simulink library "Renault_Library"  (Simulink 8.2)
settings: Solver=ode3  StartTime=0.0  StopTime=10.0  FixedStep=0.020  RelTol=1e-3
undefined symbols (not defined in the file or in its folder): ABC, AssystemProposal, b, chkval, CoeffA_C, CoeffB_C, CoeffMean_C, ConfTime_C, DiagDelay_C, DividePercent, FailureConfActive_C, FallingEdgeDuration_C, HighLevelThresholdVoltage_C, HighLevelVoltage_C, HighLevelVoltageTunable_C, InitValue_C, InputVoltRangeMax_C, InputVoltRangeMin_C, LowActiveContact_C, LowLevelThresholdVoltage_C, LowLevelVoltage_C, MaxOperatingVoltage_C, MinOperatingVoltage_C, mV2V_conversion, OUT1, OutOfRangeHigh_C, OutOfRangeLow_C, PinSupplySaturationMax, PinSupplySaturationMin, Res1Value_C, Res2Value_C, Res3Value_C, RisingEdgeDuration_C, RSA_CANSignalFailMonitoring, SampleTime_C, service14Table, service19Table, service22Table, service24Table, service28Table, service2ETable, service2FTable, service31Table, service87Table, SizeXStateDID2FTable, SizeXTable14, SizeXTable19, SizeXTable22, SizeXTable24, SizeXTable28, SizeXTable2E, SizeXTable2F, SizeXTable31, SizeXTable87, SizeYStateDID2FTable, SizeYTable14, SizeYTable19, SizeYTable22, SizeYTable24, SizeYTable28 ... (+22)
undefined symbols (not defined in the file): BatteryVoltage_ActivationThreshold_C, FrameStatus_AbsentValue_C, FrameStatus_ConfAbsentValue_C, MemThreshold_C, NAMEOFTHEMODEL_SAMPLE_TIME_CST, NbAbs1_C, NbConfAbs1_C, NbConfPres1_C, NbPres1_C, NOMDUSIGNAL_FaultConfTime_C, NOMDUSIGNAL_FaultRehabTime_C, NOMDUSIGNAL_switchvalue_C, NOMDUSIGNAL_unavailablevalue_C, PeriodFrame1_C, ResetTime_C, WakeUpTime_C
libraries: Renault_Library x112, simulink x75
(part 24 of 32)

== system Renault_Library/Services ECU/Diag/RSA_DiagnosticManagement_v02.00/DIAGNOSTIC_SANCTION_TOP/Service3E/SubFunction_In_Active_Session_Service3E ==
  SESSION : Inport
  DIAG_BUFFER_REQUEST : Inport  Port=2
  BitwiseOperator1 : simulink/Logic and Bit Operations/Bitwise Operator  logicop=AND  UseBitMask=on  BitMask=bin2dec('01111111')  BitMaskRealWorld=Stored Integer
  Constant{,4..6} (4 alike) : Constant  Value=true  OutDataTypeStr=boolean  SampleTime=-1
  Constant1 : Constant  Value=TYP_SESSION_ACTIVE.PROGRAMMING_SESSION  OutDataTypeStr=Inherit: Inherit via back propagation  SampleTime=-1
  Constant12 : Constant  Value=ZeroSubFunctionSession1  OutDataTypeStr=Inherit: Inherit via back propagation  SampleTime=-1
  Constant2 : Constant  Value=TYP_SESSION_ACTIVE.EXTENDED_SESSION  OutDataTypeStr=Inherit: Inherit via back propagation  SampleTime=-1
  Constant21 : Constant  Value=ZeroSubFunctionSession2  OutDataTypeStr=Inherit: Inherit via back propagation  SampleTime=-1
  Constant3 : Constant  Value=TYP_SESSION_ACTIVE.SAFETY_SYSTEM_SESSION  OutDataTypeStr=Inherit: Inherit via back propagation  SampleTime=-1
  Constant{7,33,48,62} (4 alike) : Constant  Value=0  OutDataTypeStr=Inherit: Inherit via back propagation  SampleTime=-1
  Constant36 : Constant  Value=ZeroSubFunctionSession3  OutDataTypeStr=Inherit: Inherit via back propagation  SampleTime=-1
  Constant50 : Constant  Value=ZeroSubFunctionSession4  OutDataTypeStr=Inherit: Inherit via back propagation  SampleTime=-1
  Constant8 : Constant  Value=TYP_SESSION_ACTIVE.DEFAULT_SESSION  OutDataTypeStr=Inherit: Inherit via back propagation  SampleTime=-1
  Logical Operator{1,6,12,17} (4 alike) : Logic  OutDataTypeStr=boolean
  LogicalOperator{,5,12,18} (4 alike) : Logic  OutDataTypeStr=boolean
  LogicalOperator24 : Logic  Operator=OR  Inputs=4  OutDataTypeStr=boolean
  RelationalOperator{1..3,7..10,23..24,33,42..43} (12 alike) : RelationalOperator  Operator===  OutDataTypeStr=boolean  InputSameDT=on
  Selector : Selector  InputPortWidth=4095  IndexOptions=Index vector (dialog)  Indices=[2]  OutputSizes=1
  SUBFUNCTION_SUPPORTED_IN_ACTIVE_SESSION : Outport
  wires:
  Constant8/1 -> RelationalOperator9/2
  SESSION/1 -> RelationalOperator9/1, RelationalOperator1/1, RelationalOperator2/1, RelationalOperator3/1
  Constant1/1 -> RelationalOperator1/2
  Constant2/1 -> RelationalOperator2/2
  Constant3/1 -> RelationalOperator3/2
  Selector/1 -> BitwiseOperator1/1
  DIAG_BUFFER_REQUEST/1 -> Selector/1
  Constant7/1 -> RelationalOperator7/2
  Constant12/1 -> RelationalOperator10/1
  Constant6/1 -> RelationalOperator10/2
  RelationalOperator10/1 -> LogicalOperator/1
  Logical Operator1/1 -> LogicalOperator/2
  RelationalOperator9/1 -> Logical Operator1/2
  RelationalOperator7/1 -> Logical Operator1/1
  Constant48/1 -> RelationalOperator33/2
  Constant36/1 -> RelationalOperator8/1
  Constant4/1 -> RelationalOperator8/2
  RelationalOperator8/1 -> LogicalOperator12/1
  Logical Operator6/1 -> LogicalOperator12/2
  RelationalOperator2/1 -> Logical Operator6/2
  RelationalOperator33/1 -> Logical Operator6/1
  Constant62/1 -> RelationalOperator42/2
  Constant50/1 -> RelationalOperator43/1
  Constant/1 -> RelationalOperator43/2
  RelationalOperator43/1 -> LogicalOperator18/1
  Logical Operator12/1 -> LogicalOperator18/2
  RelationalOperator3/1 -> Logical Operator12/2
  RelationalOperator42/1 -> Logical Operator12/1
  Constant33/1 -> RelationalOperator23/2
  Constant21/1 -> RelationalOperator24/1
  Constant5/1 -> RelationalOperator24/2
  RelationalOperator24/1 -> LogicalOperator5/1
  Logical Operator17/1 -> LogicalOperator5/2
  RelationalOperator1/1 -> Logical Operator17/2
  RelationalOperator23/1 -> Logical Operator17/1
  LogicalOperator24/1 -> SUBFUNCTION_SUPPORTED_IN_ACTIVE_SESSION/1
  BitwiseOperator1/1 -> [Number_Subfunction]
  [Number_Subfunction] -> RelationalOperator7/1
  [Number_Subfunction] -> RelationalOperator23/1
  [Number_Subfunction] -> RelationalOperator33/1
  [Number_Subfunction] -> RelationalOperator42/1
  LogicalOperator5/1 -> LogicalOperator24/2
  LogicalOperator12/1 -> LogicalOperator24/3
  LogicalOperator18/1 -> LogicalOperator24/4
  LogicalOperator/1 -> LogicalOperator24/1

== system Renault_Library/Services ECU/Diag/RSA_DiagnosticManagement_v02.00/DIAGNOSTIC_SANCTION_TOP/Service3E/SubFunction_Supported_Service3E ==
  DIAG_BUFFER_REQUEST : Inport
  BitwiseOperator1 : simulink/Logic and Bit Operations/Bitwise Operator  logicop=AND  UseBitMask=on  BitMask=bin2dec('01111111')  BitMaskRealWorld=Stored Integer
  BitwiseOperator4 : simulink/Logic and Bit Operations/Bitwise Operator  logicop=AND  UseBitMask=on  BitMask=bin2dec('10000000')  BitMaskRealWorld=Stored Integer
  Constant : Constant  Value=0  OutDataTypeStr=Inherit: Inherit via back propagation  SampleTime=-1
  Constant16 : Constant  OutDataTypeStr=Inherit: Inherit via back propagation  SampleTime=-1
  Constant3 : Constant  Value=ZeroSubFunction  OutDataTypeStr=Inherit: Inherit via back propagation  SampleTime=-1
  Constant5 : Constant  Value=true  OutDataTypeStr=boolean  SampleTime=-1
  LogicalOperator : Logic  OutDataTypeStr=boolean
  RelationalOperator : RelationalOperator  Operator===  OutDataTypeStr=boolean  InputSameDT=on
  RelationalOperator16 : RelationalOperator  Operator===  OutDataTypeStr=boolean  InputSameDT=on
  RelationalOperator4 : RelationalOperator  Operator===  OutDataTypeStr=boolean  InputSameDT=on
  Selector : Selector  InputPortWidth=4095  IndexOptions=Index vector (dialog)  Indices=[2]  OutputSizes=1
  ShiftArithmetic3 : ArithShift  BitShiftNumber=7
  SUBFUNCTION_SUPPORTED : Outport
  SUPPRESS_POS_RES : Outport  Port=2
  wires:
  DIAG_BUFFER_REQUEST/1 -> Selector/1
  Constant/1 -> RelationalOperator/2
  Constant3/1 -> RelationalOperator4/1
  Constant5/1 -> RelationalOperator4/2
  RelationalOperator/1 -> LogicalOperator/2
  RelationalOperator4/1 -> LogicalOperator/1
  RelationalOperator16/1 -> SUPPRESS_POS_RES/1
  BitwiseOperator4/1 -> ShiftArithmetic3/1
  ShiftArithmetic3/1 -> RelationalOperator16/1
  Selector/1 -> BitwiseOperator4/1, BitwiseOperator1/1
  Constant16/1 -> RelationalOperator16/2
  BitwiseOperator1/1 -> RelationalOperator/1
  LogicalOperator/1 -> SUBFUNCTION_SUPPORTED/1

== system Renault_Library/Services ECU/Diag/RSA_DiagnosticManagement_v02.00/DIAGNOSTIC_SANCTION_TOP/Service87/Check_Lenght_Message_Service87 ==
  REQ_DATA_NUMBER : Inport
  Constant1 : Constant  Value=2  OutDataTypeStr=Inherit: Inherit via back propagation  SampleTime=-1
  Constant8 : Constant  Value=6  OutDataTypeStr=Inherit: Inherit via back propagation  SampleTime=-1
  LogicalOperator : Logic  OutDataTypeStr=boolean
  RelationalOperator1 : RelationalOperator  OutDataTypeStr=boolean  InputSameDT=on
  RelationalOperator9 : RelationalOperator  Operator=<  OutDataTypeStr=boolean  InputSameDT=on
  LENGTH_MESSAGE_CORRECT : Outport
  wires:
  LogicalOperator/1 -> LENGTH_MESSAGE_CORRECT/1
  RelationalOperator1/1 -> LogicalOperator/2
  RelationalOperator9/1 -> LogicalOperator/1
  Constant1/1 -> RelationalOperator1/2
  Constant8/1 -> RelationalOperator9/2
  REQ_DATA_NUMBER/1 -> RelationalOperator1/1, RelationalOperator9/1

== system Renault_Library/Services ECU/Diag/RSA_DiagnosticManagement_v02.00/DIAGNOSTIC_SANCTION_TOP/Service87/Parameter_Valid_Service87 ==
  DIAG_BUFFER_REQUEST : Inport
  Parameter_service87 : Stateflow chart  TreatAsAtomicUnit=on
  PARAMETER_VALID : Outport
  wires:
  Parameter_service87/1(PARAMETER_VALID) -> PARAMETER_VALID/1
  DIAG_BUFFER_REQUEST/1 -> Parameter_service87/1(DIAG_BUFFER_REQUEST)

== system Renault_Library/Services ECU/Diag/RSA_DiagnosticManagement_v02.00/DIAGNOSTIC_SANCTION_TOP/Service87/SubFunction_In_Active_Session_Service87 ==
  SESSION : Inport
  DIAG_BUFFER_REQUEST : Inport  Port=2
  BitwiseOperator1 : simulink/Logic and Bit Operations/Bitwise Operator  logicop=AND  UseBitMask=on  BitMask=bin2dec('01111111')  BitMaskRealWorld=Stored Integer
  Constant1 : Constant  Value=TYP_SESSION_ACTIVE.PROGRAMMING_SESSION  OutDataTypeStr=Inherit: Inherit via back propagation  SampleTime=-1
  Constant{4..5,9..11,13,15,17..20,22} (12 alike) : Constant  Value=true  OutDataTypeStr=boolean  SampleTime=-1
  Constant12 : Constant  Value=VerifyModeTransitionWithFixedParamaterSession1  OutDataTypeStr=Inherit: Inherit via back propagation  SampleTime=-1
  Constant14 : Constant  Value=VerifyModeTransitionWithSpecificParamaterSession1  OutDataTypeStr=Inherit: Inherit via back propagation  SampleTime=-1
  Constant16 : Constant  Value=TransitionModeSession1  OutDataTypeStr=Inherit: Inherit via back propagation  SampleTime=-1
  Constant2 : Constant  Value=TYP_SESSION_ACTIVE.EXTENDED_SESSION  OutDataTypeStr=Inherit: Inherit via back propagation  SampleTime=-1
  Constant21 : Constant  Value=VerifyModeTransitionWithFixedParamaterSession2  OutDataTypeStr=Inherit: Inherit via back propagation  SampleTime=-1
  Constant23 : Constant  Value=VerifyModeTransitionWithSpecificParamaterSession2  OutDataTypeStr=Inherit: Inherit via back propagation  SampleTime=-1
  Constant25 : Constant  Value=TransitionModeSession2  OutDataTypeStr=Inherit: Inherit via back propagation  SampleTime=-1
  Constant3 : Constant  Value=TYP_SESSION_ACTIVE.SAFETY_SYSTEM_SESSION  OutDataTypeStr=Inherit: Inherit via back propagation  SampleTime=-1
  Constant{6,31,46,60} (4 alike) : Constant  Value=3  OutDataTypeStr=Inherit: Inherit via back propagation  SampleTime=-1
  Constant{32,47,61,65} (4 alike) : Constant  Value=2  OutDataTypeStr=Inherit: Inherit via back propagation  SampleTime=-1
  Constant{7,33,48,62} (4 alike) : Constant  OutDataTypeStr=Inherit: Inherit via back propagation  SampleTime=-1
  Constant36 : Constant  Value=VerifyModeTransitionWithFixedParamaterSession3  OutDataTypeStr=Inherit: Inherit via back propagation  SampleTime=-1
  Constant38 : Constant  Value=VerifyModeTransitionWithSpecificParamaterSession3  OutDataTypeStr=Inherit: Inherit via back propagation  SampleTime=-1
  Constant40 : Constant  Value=TransitionModeSession3  OutDataTypeStr=Inherit: Inherit via back propagation  SampleTime=-1
  Constant50 : Constant  Value=VerifyModeTransitionWithFixedParamaterSession4  OutDataTypeStr=Inherit: Inherit via back propagation  SampleTime=-1
  Constant52 : Constant  Value=VerifyModeTransitionWithSpecificParamaterSession4  OutDataTypeStr=Inherit: Inherit via back propagation  SampleTime=-1
  Constant54 : Constant  Value=TransitionModeSession4  OutDataTypeStr=Inherit: Inherit via back propagation  SampleTime=-1
  Constant8 : Constant  Value=TYP_SESSION_ACTIVE.DEFAULT_SESSION  OutDataTypeStr=Inherit: Inherit via back propagation  SampleTime=-1
  Logical Operator{1..2,5..7,10..13,16..18} (12 alike) : Logic  OutDataTypeStr=boolean
  LogicalOperator{,1..2,5,7..8,12..14,18..20} (12 alike) : Logic  OutDataTypeStr=boolean
  LogicalOperator{4,10,16,22} (4 alike) : Logic  Operator=OR  Inputs=3  OutDataTypeStr=boolean
  LogicalOperator24 : Logic  Operator=OR  Inputs=4  OutDataTypeStr=boolean
  RelationalOperator{1..3,6..12,15..16,20,22..26,30,32..35,39,41..43,45} (28 alike) : RelationalOperator  Operator===  OutDataTypeStr=boolean  InputSameDT=on
  Selector : Selector  InputPortWidth=4095  IndexOptions=Index vector (dialog)  Indices=[2]  OutputSizes=1
  SUBFUNCTION_SUPPORTED_IN_ACTIVE_SESSION : Outport
  wires:
  [Number_Subfunction] -> RelationalOperator41/1
  [Number_Subfunction] -> RelationalOperator39/1
  [Number_Subfunction] -> RelationalOperator42/1
  [Number_Subfunction] -> RelationalOperator32/1
  [Number_Subfunction] -> RelationalOperator30/1
  [Number_Subfunction] -> RelationalOperator33/1
  [Number_Subfunction] -> RelationalOperator22/1
  [Number_Subfunction] -> RelationalOperator20/1
  [Number_Subfunction] -> RelationalOperator23/1
  [Number_Subfunction] -> RelationalOperator6/1
  [Number_Subfunction] -> RelationalOperator45/1
  [Number_Subfunction] -> RelationalOperator7/1
  BitwiseOperator1/1 -> [Number_Subfunction]
  LogicalOperator24/1 -> SUBFUNCTION_SUPPORTED_IN_ACTIVE_SESSION/1
  LogicalOperator4/1 -> LogicalOperator24/1
  LogicalOperator22/1 -> LogicalOperator24/4
  LogicalOperator16/1 -> LogicalOperator24/3
  LogicalOperator10/1 -> LogicalOperator24/2
  RelationalOperator22/1 -> Logical Operator16/1
  RelationalOperator23/1 -> Logical Operator17/1
  Logical Operator16/1 -> LogicalOperator8/2
  Constant31/1 -> RelationalOperator22/2
  RelationalOperator20/1 -> Logical Operator18/1
  Constant32/1 -> RelationalOperator20/2
  RelationalOperator1/1 -> Logical Operator16/2, Logical Operator18/2, Logical Operator17/2
  Logical Operator17/1 -> LogicalOperator5/2
  Logical Operator18/1 -> LogicalOperator7/2
  LogicalOperator5/1 -> LogicalOperator10/1
  LogicalOperator8/1 -> LogicalOperator10/3
  LogicalOperator7/1 -> LogicalOperator10/2
  RelationalOperator16/1 -> LogicalOperator8/1
  Constant25/1 -> RelationalOperator16/1
  Constant13/1 -> RelationalOperator16/2
  RelationalOperator15/1 -> LogicalOperator7/1
  Constant23/1 -> RelationalOperator15/1
  Constant11/1 -> RelationalOperator15/2
  RelationalOperator24/1 -> LogicalOperator5/1
  Constant10/1 -> RelationalOperator24/2
  Constant21/1 -> RelationalOperator24/1
  Constant33/1 -> RelationalOperator23/2
  RelationalOperator41/1 -> Logical Operator11/1
  RelationalOperator42/1 -> Logical Operator12/1
  Logical Operator11/1 -> LogicalOperator20/2
  Constant60/1 -> RelationalOperator41/2
  RelationalOperator39/1 -> Logical Operator13/1
  Constant61/1 -> RelationalOperator39/2
  RelationalOperator3/1 -> Logical Operator11/2, Logical Operator13/2, Logical Operator12/2
  Logical Operator12/1 -> LogicalOperator18/2
  Logical Operator13/1 -> LogicalOperator19/2
  LogicalOperator18/1 -> LogicalOperator22/1
  LogicalOperator20/1 -> LogicalOperator22/3
  LogicalOperator19/1 -> LogicalOperator22/2
  RelationalOperator35/1 -> LogicalOperator20/1
  Constant54/1 -> RelationalOperator35/1
  Constant22/1 -> RelationalOperator35/2
  RelationalOperator34/1 -> LogicalOperator19/1
  Constant52/1 -> RelationalOperator34/1
  Constant20/1 -> RelationalOperator34/2
  RelationalOperator43/1 -> LogicalOperator18/1
  Constant19/1 -> RelationalOperator43/2
  Constant50/1 -> RelationalOperator43/1
  Constant62/1 -> RelationalOperator42/2
  RelationalOperator32/1 -> Logical Operator10/1
  RelationalOperator33/1 -> Logical Operator6/1
  Logical Operator10/1 -> LogicalOperator14/2
  Constant46/1 -> RelationalOperator32/2
  RelationalOperator30/1 -> Logical Operator7/1
  Constant47/1 -> RelationalOperator30/2
  RelationalOperator2/1 -> Logical Operator10/2, Logical Operator7/2, Logical Operator6/2
  Logical Operator6/1 -> LogicalOperator12/2
  Logical Operator7/1 -> LogicalOperator13/2
  LogicalOperator12/1 -> LogicalOperator16/1
  LogicalOperator14/1 -> LogicalOperator16/3
  LogicalOperator13/1 -> LogicalOperator16/2
  RelationalOperator26/1 -> LogicalOperator14/1
  Constant40/1 -> RelationalOperator26/1
  Constant18/1 -> RelationalOperator26/2
  RelationalOperator25/1 -> LogicalOperator13/1
  Constant38/1 -> RelationalOperator25/1
  Constant17/1 -> RelationalOperator25/2
  RelationalOperator8/1 -> LogicalOperator12/1
  Constant15/1 -> RelationalOperator8/2
  Constant36/1 -> RelationalOperator8/1
  Constant48/1 -> RelationalOperator33/2
  RelationalOperator6/1 -> Logical Operator5/1
  RelationalOperator7/1 -> Logical Operator1/1
  Logical Operator5/1 -> LogicalOperator2/2
  Constant6/1 -> RelationalOperator6/2
  RelationalOperator45/1 -> Logical Operator2/1
  Constant65/1 -> RelationalOperator45/2
  RelationalOperator9/1 -> Logical Operator5/2, Logical Operator2/2, Logical Operator1/2
  Logical Operator1/1 -> LogicalOperator/2
  Logical Operator2/1 -> LogicalOperator1/2
  LogicalOperator/1 -> LogicalOperator4/1
  LogicalOperator2/1 -> LogicalOperator4/3
  LogicalOperator1/1 -> LogicalOperator4/2
  RelationalOperator12/1 -> LogicalOperator2/1
  Constant16/1 -> RelationalOperator12/1
  Constant9/1 -> RelationalOperator12/2
  RelationalOperator11/1 -> LogicalOperator1/1
  Constant14/1 -> RelationalOperator11/1
  Constant5/1 -> RelationalOperator11/2
  RelationalOperator10/1 -> LogicalOperator/1
  Constant4/1 -> RelationalOperator10/2
  Constant12/1 -> RelationalOperator10/1
  Constant7/1 -> RelationalOperator7/2
  DIAG_BUFFER_REQUEST/1 -> Selector/1
  Selector/1 -> BitwiseOperator1/1
  Constant3/1 -> RelationalOperator3/2
  Constant2/1 -> RelationalOperator2/2
  Constant1/1 -> RelationalOperator1/2
  SESSION/1 -> RelationalOperator3/1, RelationalOperator2/1, RelationalOperator1/1, RelationalOperator9/1
  Constant8/1 -> RelationalOperator9/2

== system Renault_Library/Services ECU/Diag/RSA_DiagnosticManagement_v02.00/DIAGNOSTIC_SANCTION_TOP/Service87/SubFunction_Supported_Service87 ==
  DIAG_BUFFER_REQUEST : Inport
  BitwiseOperator1 : simulink/Logic and Bit Operations/Bitwise Operator  logicop=AND  UseBitMask=on  BitMask=bin2dec('01111111')  BitMaskRealWorld=Stored Integer
  BitwiseOperator4 : simulink/Logic and Bit Operations/Bitwise Operator  logicop=AND  UseBitMask=on  BitMask=bin2dec('10000000')  BitMaskRealWorld=Stored Integer
  Constant1 : Constant  OutDataTypeStr=Inherit: Inherit via back propagation  SampleTime=-1
  Constant10 : Constant  Value=TransitionMode  OutDataTypeStr=Inherit: Inherit via back propagation  SampleTime=-1
  Constant16 : Constant  OutDataTypeStr=Inherit: Inherit via back propagation  SampleTime=-1
  Constant2 : Constant  Value=2  OutDataTypeStr=Inherit: Inherit via back propagation  SampleTime=-1
  Constant3 : Constant  Value=true  OutDataTypeStr=boolean  SampleTime=-1
  Constant4 : Constant  Value=3  OutDataTypeStr=Inherit: Inherit via back propagation  SampleTime=-1
  Constant5 : Constant  Value=true  OutDataTypeStr=boolean  SampleTime=-1
  Constant6 : Constant  Value=VerifyModeTransitionWithFixedParamater  OutDataTypeStr=Inherit: Inherit via back propagation  SampleTime=-1
  Constant7 : Constant  Value=true  OutDataTypeStr=boolean  SampleTime=-1
  Constant8 : Constant  Value=VerifyModeTransitionWithSpecificParamater  OutDataTypeStr=Inherit: Inherit via back propagation  SampleTime=-1
  LogicalOperator1 : Logic  OutDataTypeStr=boolean
  LogicalOperator2 : Logic  OutDataTypeStr=boolean
  LogicalOperator3 : Logic  OutDataTypeStr=boolean
  LogicalOperator4 : Logic  Operator=OR  Inputs=3  OutDataTypeStr=boolean
  RelationalOperator{1..3,5..7,16} (7 alike) : RelationalOperator  Operator===  OutDataTypeStr=boolean  InputSameDT=on
  Selector : Selector  InputPortWidth=4095  IndexOptions=Index vector (dialog)  Indices=[2]  OutputSizes=1
  ShiftArithmetic3 : ArithShift  BitShiftNumber=7
  SUBFUNCTION_SUPPORTED : Outport
  SUPPRESS_POS_RES : Outport  Port=2
  wires:
  LogicalOperator3/1 -> LogicalOperator4/3
  LogicalOperator2/1 -> LogicalOperator4/2
  LogicalOperator1/1 -> LogicalOperator4/1
  BitwiseOperator1/1 -> RelationalOperator3/1, RelationalOperator2/1, RelationalOperator1/1
  Constant16/1 -> RelationalOperator16/2
  Selector/1 -> BitwiseOperator1/1, BitwiseOperator4/1
  ShiftArithmetic3/1 -> RelationalOperator16/1
  BitwiseOperator4/1 -> ShiftArithmetic3/1
  RelationalOperator16/1 -> SUPPRESS_POS_RES/1
  RelationalOperator3/1 -> LogicalOperator3/2
  LogicalOperator4/1 -> SUBFUNCTION_SUPPORTED/1
  RelationalOperator7/1 -> LogicalOperator3/1
  Constant7/1 -> RelationalOperator7/2
  Constant10/1 -> RelationalOperator7/1
  RelationalOperator6/1 -> LogicalOperator2/1
  RelationalOperator2/1 -> LogicalOperator2/2
  Constant8/1 -> RelationalOperator6/1
  Constant5/1 -> RelationalOperator6/2
  RelationalOperator5/1 -> LogicalOperator1/1
  RelationalOperator1/1 -> LogicalOperator1/2
  Constant6/1 -> RelationalOperator5/1
  Constant3/1 -> RelationalOperator5/2
  Constant4/1 -> RelationalOperator3/2
  Constant2/1 -> RelationalOperator2/2
  Constant1/1 -> RelationalOperator1/2
  DIAG_BUFFER_REQUEST/1 -> Selector/1

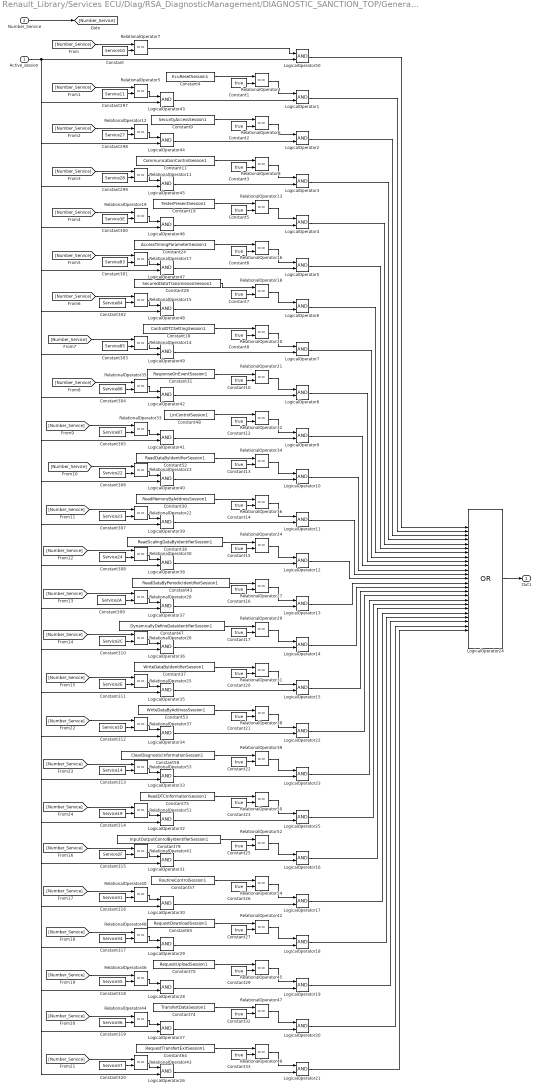
== system Renault_Library/Services ECU/Diag/RSA_DiagnosticManagement/DIAGNOSTIC_SANCTION_TOP/GeneralService/Service_Supported_In_Active_Session/SubsystemDefaultSession ==
  Active_session : Inport
  Number_Service : Inport  Port=2  OutDataTypeStr=uint8
  Constant : Constant  Value=Service10  OutDataTypeStr=Inherit: Inherit via back propagation  SampleTime=-1
  Constant{1..3,5..8,10,12..17,20..23,25..27,29,32..33} (24 alike) : Constant  Value=true  OutDataTypeStr=boolean  SampleTime=-1
  Constant11 : Constant  Value=CommunicationControlSession1  OutDataTypeStr=Inherit: Inherit via back propagation  SampleTime=-1
  Constant18 : Constant  Value=ControlDTCSettingSession1  OutDataTypeStr=Inherit: Inherit via back propagation  SampleTime=-1
  Constant19 : Constant  Value=TesterPresentSession1  OutDataTypeStr=Inherit: Inherit via back propagation  SampleTime=-1
  Constant24 : Constant  Value=AccessTimingParameterSession1  OutDataTypeStr=Inherit: Inherit via back propagation  SampleTime=-1
  Constant28 : Constant  Value=SecuredDataTransmissionSession1  OutDataTypeStr=Inherit: Inherit via back propagation  SampleTime=-1
  Constant297 : Constant  Value=Service11  OutDataTypeStr=Inherit: Inherit via back propagation  SampleTime=-1
  Constant298 : Constant  Value=Service27  OutDataTypeStr=Inherit: Inherit via back propagation  SampleTime=-1
  Constant299 : Constant  Value=Service28  OutDataTypeStr=Inherit: Inherit via back propagation  SampleTime=-1
  Constant30 : Constant  Value=ReadMemoryByAddressSession1  OutDataTypeStr=Inherit: Inherit via back propagation  SampleTime=-1
  Constant300 : Constant  Value=Service3E  OutDataTypeStr=Inherit: Inherit via back propagation  SampleTime=-1
  Constant301 : Constant  Value=Service83  OutDataTypeStr=Inherit: Inherit via back propagation  SampleTime=-1
  Constant302 : Constant  Value=Service84  OutDataTypeStr=Inherit: Inherit via back propagation  SampleTime=-1
  Constant303 : Constant  Value=Service85  OutDataTypeStr=Inherit: Inherit via back propagation  SampleTime=-1
  Constant304 : Constant  Value=Service86  OutDataTypeStr=Inherit: Inherit via back propagation  SampleTime=-1
  Constant305 : Constant  Value=Service87  OutDataTypeStr=Inherit: Inherit via back propagation  SampleTime=-1
  Constant306 : Constant  Value=Service22  OutDataTypeStr=Inherit: Inherit via back propagation  SampleTime=-1
  Constant307 : Constant  Value=Service23  OutDataTypeStr=Inherit: Inherit via back propagation  SampleTime=-1
  Constant308 : Constant  Value=Service24  OutDataTypeStr=Inherit: Inherit via back propagation  SampleTime=-1
  Constant309 : Constant  Value=Service2A  OutDataTypeStr=Inherit: Inherit via back propagation  SampleTime=-1
  Constant31 : Constant  Value=ResponseOnEventSession1  OutDataTypeStr=Inherit: Inherit via back propagation  SampleTime=-1
  Constant310 : Constant  Value=Service2C  OutDataTypeStr=Inherit: Inherit via back propagation  SampleTime=-1
  Constant311 : Constant  Value=Service2E  OutDataTypeStr=Inherit: Inherit via back propagation  SampleTime=-1
  Constant312 : Constant  Value=Service3D  OutDataTypeStr=Inherit: Inherit via back propagation  SampleTime=-1
  Constant313 : Constant  Value=Service14  OutDataTypeStr=Inherit: Inherit via back propagation  SampleTime=-1
  Constant314 : Constant  Value=Service19  OutDataTypeStr=Inherit: Inherit via back propagation  SampleTime=-1
  Constant315 : Constant  Value=Service2F  OutDataTypeStr=Inherit: Inherit via back propagation  SampleTime=-1
  Constant316 : Constant  Value=Service31  OutDataTypeStr=Inherit: Inherit via back propagation  SampleTime=-1
  Constant317 : Constant  Value=Service34  OutDataTypeStr=Inherit: Inherit via back propagation  SampleTime=-1
  Constant318 : Constant  Value=Service35  OutDataTypeStr=Inherit: Inherit via back propagation  SampleTime=-1
  Constant319 : Constant  Value=Service36  OutDataTypeStr=Inherit: Inherit via back propagation  SampleTime=-1
  Constant320 : Constant  Value=Service37  OutDataTypeStr=Inherit: Inherit via back propagation  SampleTime=-1
  Constant37 : Constant  Value=WriteDataByIdentifierSession1  OutDataTypeStr=Inherit: Inherit via back propagation  SampleTime=-1
  Constant38 : Constant  Value=ReadScalingDataByIdentifierSession1  OutDataTypeStr=Inherit: Inherit via back propagation  SampleTime=-1
  Constant4 : Constant  Value=EcuResetSession1  OutDataTypeStr=Inherit: Inherit via back propagation  SampleTime=-1
  Constant43 : Constant  Value=ReadDataByPeriodicIdentifierSession1  OutDataTypeStr=Inherit: Inherit via back propagation  SampleTime=-1
  Constant47 : Constant  Value=DynamicallyDefineDataIdentifierSession1  OutDataTypeStr=Inherit: Inherit via back propagation  SampleTime=-1
  Constant48 : Constant  Value=LinControlSession1  OutDataTypeStr=Inherit: Inherit via back propagation  SampleTime=-1
  Constant52 : Constant  Value=ReadDataByIdentifierSession1  OutDataTypeStr=Inherit: Inherit via back propagation  SampleTime=-1
  Constant53 : Constant  Value=WriteDataByAddressSession1  OutDataTypeStr=Inherit: Inherit via back propagation  SampleTime=-1
  Constant57 : Constant  Value=RoutineControlSession1  OutDataTypeStr=Inherit: Inherit via back propagation  SampleTime=-1
  Constant58 : Constant  Value=ClearDiagnosticInformationSession1  OutDataTypeStr=Inherit: Inherit via back propagation  SampleTime=-1
  Constant64 : Constant  Value=RequestTransfertExitSession1  OutDataTypeStr=Inherit: Inherit via back propagation  SampleTime=-1
  Constant65 : Constant  Value=RequestDownloadSession1  OutDataTypeStr=Inherit: Inherit via back propagation  SampleTime=-1
  Constant70 : Constant  Value=RequestUploadSession1  OutDataTypeStr=Inherit: Inherit via back propagation  SampleTime=-1
  Constant74 : Constant  Value=TransfertDataSession1  OutDataTypeStr=Inherit: Inherit via back propagation  SampleTime=-1
  Constant75 : Constant  Value=ReadDTCInformationSession1  OutDataTypeStr=Inherit: Inherit via back propagation  SampleTime=-1
  Constant79 : Constant  Value=InputOutputConrolByIdentifierSession1  OutDataTypeStr=Inherit: Inherit via back propagation  SampleTime=-1
  Constant9 : Constant  Value=SecurityAccessSession1  OutDataTypeStr=Inherit: Inherit via back propagation  SampleTime=-1
  LogicalOperator{1..23,25..50} (49 alike) : Logic  OutDataTypeStr=boolean
  LogicalOperator24 : Logic  Operator=OR  Inputs=25  OutDataTypeStr=boolean
  RelationalOperator{4..8,11..54} (49 alike) : RelationalOperator  Operator===  OutDataTypeStr=boolean  InputSameDT=on
  Out1 : Outport
  wires:
  Constant/1 -> RelationalOperator7/2
  Active_session/1 -> LogicalOperator33/2, LogicalOperator32/2, LogicalOperator31/2, LogicalOperator30/2, LogicalOperator29/2, LogicalOperator28/2, LogicalOperator27/2, LogicalOperator26/2, LogicalOperator34/2, LogicalOperator40/2, LogicalOperator39/2, LogicalOperator38/2, LogicalOperator37/2, LogicalOperator36/2, LogicalOperator35/2, LogicalOperator41/2, LogicalOperator42/2, LogicalOperator49/2, LogicalOperator48/2, LogicalOperator47/2, LogicalOperator46/2, LogicalOperator45/2, LogicalOperator44/2, LogicalOperator43/2, LogicalOperator50/2
  RelationalOperator7/1 -> LogicalOperator50/1
  [Number_Service] -> RelationalOperator7/1
  Constant297/1 -> RelationalOperator5/2
  Constant4/1 -> RelationalOperator4/1
  Constant1/1 -> RelationalOperator4/2
  RelationalOperator4/1 -> LogicalOperator1/1
  LogicalOperator43/1 -> LogicalOperator1/2
  RelationalOperator5/1 -> LogicalOperator43/1
  [Number_Service] -> RelationalOperator5/1
  Constant298/1 -> RelationalOperator12/2
  Constant9/1 -> RelationalOperator6/1
  Constant2/1 -> RelationalOperator6/2
  RelationalOperator6/1 -> LogicalOperator2/1
  LogicalOperator44/1 -> LogicalOperator2/2
  RelationalOperator12/1 -> LogicalOperator44/1
  [Number_Service] -> RelationalOperator12/1
  Constant299/1 -> RelationalOperator11/2
  Constant11/1 -> RelationalOperator8/1
  Constant3/1 -> RelationalOperator8/2
  RelationalOperator8/1 -> LogicalOperator3/1
  LogicalOperator45/1 -> LogicalOperator3/2
  RelationalOperator11/1 -> LogicalOperator45/1
  [Number_Service] -> RelationalOperator11/1
  Constant300/1 -> RelationalOperator19/2
  Constant19/1 -> RelationalOperator13/1
  Constant5/1 -> RelationalOperator13/2
  RelationalOperator13/1 -> LogicalOperator4/1
  LogicalOperator46/1 -> LogicalOperator4/2
  RelationalOperator19/1 -> LogicalOperator46/1
  [Number_Service] -> RelationalOperator19/1
  Constant301/1 -> RelationalOperator17/2
  Constant24/1 -> RelationalOperator16/1
  Constant6/1 -> RelationalOperator16/2
  RelationalOperator16/1 -> LogicalOperator5/1
  LogicalOperator47/1 -> LogicalOperator5/2
  RelationalOperator17/1 -> LogicalOperator47/1
  [Number_Service] -> RelationalOperator17/1
  Constant302/1 -> RelationalOperator15/2
  Constant28/1 -> RelationalOperator18/1
  Constant7/1 -> RelationalOperator18/2
  RelationalOperator18/1 -> LogicalOperator6/1
  LogicalOperator48/1 -> LogicalOperator6/2
  RelationalOperator15/1 -> LogicalOperator48/1
  [Number_Service] -> RelationalOperator15/1
  Constant303/1 -> RelationalOperator14/2
  Constant18/1 -> RelationalOperator20/1
  Constant8/1 -> RelationalOperator20/2
  RelationalOperator20/1 -> LogicalOperator7/1
  LogicalOperator49/1 -> LogicalOperator7/2
  RelationalOperator14/1 -> LogicalOperator49/1
  [Number_Service] -> RelationalOperator14/1
  Constant304/1 -> RelationalOperator35/2
  Constant31/1 -> RelationalOperator21/1
  Constant10/1 -> RelationalOperator21/2
  RelationalOperator21/1 -> LogicalOperator8/1
  LogicalOperator42/1 -> LogicalOperator8/2
  RelationalOperator35/1 -> LogicalOperator42/1
  [Number_Service] -> RelationalOperator35/1
  Constant305/1 -> RelationalOperator33/2
  Constant48/1 -> RelationalOperator32/1
  Constant12/1 -> RelationalOperator32/2
  RelationalOperator32/1 -> LogicalOperator9/1
  LogicalOperator41/1 -> LogicalOperator9/2
  RelationalOperator33/1 -> LogicalOperator41/1
  [Number_Service] -> RelationalOperator33/1
  Constant306/1 -> RelationalOperator23/2
  Constant52/1 -> RelationalOperator34/1
  Constant13/1 -> RelationalOperator34/2
  RelationalOperator34/1 -> LogicalOperator10/1
  LogicalOperator40/1 -> LogicalOperator10/2
  RelationalOperator23/1 -> LogicalOperator40/1
  [Number_Service] -> RelationalOperator23/1
  Constant307/1 -> RelationalOperator22/2
  Constant30/1 -> RelationalOperator36/1
  Constant14/1 -> RelationalOperator36/2
  RelationalOperator36/1 -> LogicalOperator11/1
  LogicalOperator39/1 -> LogicalOperator11/2
  RelationalOperator22/1 -> LogicalOperator39/1
  [Number_Service] -> RelationalOperator22/1
  Constant308/1 -> RelationalOperator30/2
  Constant38/1 -> RelationalOperator24/1
  Constant15/1 -> RelationalOperator24/2
  RelationalOperator24/1 -> LogicalOperator12/1
  LogicalOperator38/1 -> LogicalOperator12/2
  RelationalOperator30/1 -> LogicalOperator38/1
  [Number_Service] -> RelationalOperator30/1
  Constant309/1 -> RelationalOperator28/2
  Constant43/1 -> RelationalOperator27/1
  Constant16/1 -> RelationalOperator27/2
  RelationalOperator27/1 -> LogicalOperator13/1
  LogicalOperator37/1 -> LogicalOperator13/2
  RelationalOperator28/1 -> LogicalOperator37/1
  [Number_Service] -> RelationalOperator28/1
  Constant310/1 -> RelationalOperator26/2
  Constant47/1 -> RelationalOperator29/1
  Constant17/1 -> RelationalOperator29/2
  RelationalOperator29/1 -> LogicalOperator14/1
  LogicalOperator36/1 -> LogicalOperator14/2
  RelationalOperator26/1 -> LogicalOperator36/1
  [Number_Service] -> RelationalOperator26/1
  Constant311/1 -> RelationalOperator25/2
  Constant37/1 -> RelationalOperator31/1
  Constant20/1 -> RelationalOperator31/2
  RelationalOperator31/1 -> LogicalOperator15/1
  LogicalOperator35/1 -> LogicalOperator15/2
  RelationalOperator25/1 -> LogicalOperator35/1
  [Number_Service] -> RelationalOperator25/1
  Constant312/1 -> RelationalOperator37/2
  Constant53/1 -> RelationalOperator38/1
  Constant21/1 -> RelationalOperator38/2
  RelationalOperator38/1 -> LogicalOperator22/1
  LogicalOperator34/1 -> LogicalOperator22/2
  RelationalOperator37/1 -> LogicalOperator34/1
  [Number_Service] -> RelationalOperator37/1
  Constant313/1 -> RelationalOperator53/2
  Constant58/1 -> RelationalOperator39/1
  Constant22/1 -> RelationalOperator39/2
  RelationalOperator39/1 -> LogicalOperator23/1
  LogicalOperator33/1 -> LogicalOperator23/2
  RelationalOperator53/1 -> LogicalOperator33/1
  [Number_Service] -> RelationalOperator53/1
  Constant314/1 -> RelationalOperator51/2
  Constant75/1 -> RelationalOperator50/1
  Constant23/1 -> RelationalOperator50/2
  RelationalOperator50/1 -> LogicalOperator25/1
  LogicalOperator32/1 -> LogicalOperator25/2
  RelationalOperator51/1 -> LogicalOperator32/1
  [Number_Service] -> RelationalOperator51/1
  Constant315/1 -> RelationalOperator41/2
  Constant79/1 -> RelationalOperator52/1
  Constant25/1 -> RelationalOperator52/2
  RelationalOperator52/1 -> LogicalOperator16/1
  LogicalOperator31/1 -> LogicalOperator16/2
  RelationalOperator41/1 -> LogicalOperator31/1
  [Number_Service] -> RelationalOperator41/1
  Constant316/1 -> RelationalOperator40/2
  Constant57/1 -> RelationalOperator54/1
  Constant26/1 -> RelationalOperator54/2
  RelationalOperator54/1 -> LogicalOperator17/1
  LogicalOperator30/1 -> LogicalOperator17/2
  RelationalOperator40/1 -> LogicalOperator30/1
  [Number_Service] -> RelationalOperator40/1
  Constant317/1 -> RelationalOperator48/2
  Constant65/1 -> RelationalOperator42/1
  Constant27/1 -> RelationalOperator42/2
  RelationalOperator42/1 -> LogicalOperator18/1
  LogicalOperator29/1 -> LogicalOperator18/2
  RelationalOperator48/1 -> LogicalOperator29/1
  [Number_Service] -> RelationalOperator48/1
  Constant318/1 -> RelationalOperator46/2
  Constant70/1 -> RelationalOperator45/1
  Constant29/1 -> RelationalOperator45/2
  RelationalOperator45/1 -> LogicalOperator19/1
  LogicalOperator28/1 -> LogicalOperator19/2
  RelationalOperator46/1 -> LogicalOperator28/1
  [Number_Service] -> RelationalOperator46/1
  Constant319/1 -> RelationalOperator44/2
  Constant74/1 -> RelationalOperator47/1
  Constant32/1 -> RelationalOperator47/2
  RelationalOperator47/1 -> LogicalOperator20/1
  LogicalOperator27/1 -> LogicalOperator20/2
  RelationalOperator44/1 -> LogicalOperator27/1
  [Number_Service] -> RelationalOperator44/1
  Constant320/1 -> RelationalOperator43/2
  Constant64/1 -> RelationalOperator49/1
  Constant33/1 -> RelationalOperator49/2
  RelationalOperator49/1 -> LogicalOperator21/1
  LogicalOperator26/1 -> LogicalOperator21/2
  RelationalOperator43/1 -> LogicalOperator26/1
  [Number_Service] -> RelationalOperator43/1
  LogicalOperator50/1 -> LogicalOperator24/1
  LogicalOperator1/1 -> LogicalOperator24/2
  LogicalOperator2/1 -> LogicalOperator24/3
  LogicalOperator3/1 -> LogicalOperator24/4
  LogicalOperator4/1 -> LogicalOperator24/5
  LogicalOperator5/1 -> LogicalOperator24/6
  LogicalOperator6/1 -> LogicalOperator24/7
  LogicalOperator7/1 -> LogicalOperator24/8
  LogicalOperator8/1 -> LogicalOperator24/9
  LogicalOperator9/1 -> LogicalOperator24/10
  LogicalOperator10/1 -> LogicalOperator24/11
  LogicalOperator11/1 -> LogicalOperator24/12
  LogicalOperator12/1 -> LogicalOperator24/13
  LogicalOperator13/1 -> LogicalOperator24/14
  LogicalOperator14/1 -> LogicalOperator24/15
  LogicalOperator15/1 -> LogicalOperator24/16
  LogicalOperator22/1 -> LogicalOperator24/17
  LogicalOperator23/1 -> LogicalOperator24/18
  LogicalOperator25/1 -> LogicalOperator24/19
  LogicalOperator16/1 -> LogicalOperator24/20
  LogicalOperator17/1 -> LogicalOperator24/21
  LogicalOperator18/1 -> LogicalOperator24/22
  LogicalOperator19/1 -> LogicalOperator24/23
  LogicalOperator20/1 -> LogicalOperator24/24
  LogicalOperator21/1 -> LogicalOperator24/25
  LogicalOperator24/1 -> Out1/1
  Number_Service/1 -> [Number_Service]
